simulink model "sim_discrete_planar_2dof"  (R2022b)
settings: Solver=VariableStepAuto  StartTime=0.0  StopTime=T_sim  FixedStep=param_global.Ta  RelTol=1e-3
InitFcn:
  | parameters_2dof;
undefined symbols (not defined in the file): coord_xaxis_len, coord_yaxis_len, coord_zaxis_len, fx, fy, fz, init_bus_param, param_global, param_path_set_points, param_robot, param_vis, path_coord_xaxis_len, path_coord_yaxis_len, path_coord_zaxis_len, path_param, q_0, q_0_p, tau_x, tau_y, tau_z, X, Y, Z
libraries: sm_lib x1241, nesl_utility x796
(part 34 of 34)

== matlab function sim_discrete_planar_2dof/Visualization q2/Spur Endeffektor/MATLAB Function ==
  settings: updateMethod=DISCRETE  sampleTime=param_global.Ta
  data: robot_model input; p1 output; p2 output; p3 output; p4 output; p5 output; p6 output; p7 output; p8 output; p9 output; p10 output; p11 output; p12 output; p13 output; p14 output; p15 output; p16 output; p17 output; p18 output; p19 output; p20 output; p21 output; p22 output; p23 output; p24 output; p25 output; p26 output; p27 output; p28 output; p29 output; p30 output; p31 output; p32 output; p33 output; p34 output; p35 output; p36 output; p37 output; p38 output; p39 output; p40 output; p41 output; p42 output; p43 output; p44 output; p45 output; p46 output; p47 output; p48 output; p49 output; p50 output; start input; delta input; p51 output; p52 output; p53 output; p54 output; p55 output; p56 output; p57 output; p58 output; p59 output; p60 output; p61 output; p62 output; p63 output; p64 output; p65 output; p66 output; p67 output; p68 output; p69 output; p70 output; p71 output; p72 output; p73 output; p74 output; p75 output; p76 output; p77 output; p78 output; p79 output; p80 output; p81 output; p82 output; p83 output; p84 output; p85 output; p86 output; p87 output; p88 output; p89 output; p90 output; p91 output; p92 output; p93 output; p94 output; p95 output; p96 output; p97 output; p98 output; p99 output; p100 output
function [p1,p2,p3,p4,p5,p6,p7,p8,p9,p10,p11,p12,p13,p14,p15,p16,p17,p18,p19,p20,p21,p22,p23,p24,p25,p26,p27,p28,p29,p30,p31,p32,p33,p34,p35,p36,p37,p38,p39,p40,p41,p42,p43,p44,p45,p46,p47,p48,p49,p50,p51,p52,p53,p54,p55,p56,p57,p58,p59,p60,p61,p62,p63,p64,p65,p66,p67,p68,p69,p70,p71,p72,p73,p74,p75,p76,p77,p78,p79,p80,p81,p82,p83,p84,p85,p86,p87,p88,p89,p90,p91,p92,p93,p94,p95,p96,p97,p98,p99,p100] = fcn(robot_model, start, delta)
persistent i t1 t2 t3 t4 t5 t6 t7 t8 t9 t10 t11 t12 t13 t14 t15 t16 t17 t18 t19 t20 t21 t22 t23 t24 t25 t26 t27 t28 t29 t30 t31 t32 t33 t34 t35 t36 t37 t38 t39 t40 t41 t42 t43 t44 t45 t46 t47 t48 t49 t50 t51 t52 t53 t54 t55 t56 t57 t58 t59 t60 t61 t62 t63 t64 t65 t66 t67 t68 t69 t70 t71 t72 t73 t74 t75 t76 t77 t78 t79 t80 t81 t82 t83 t84 t85 t86 t87 t88 t89 t90 t91 t92 t93 t94 t95 t96 t97 t98 t99 t100
if isempty(t1)
    i = 1;
    t1 = robot_model.H(1:3,4);
    t2 = t1;
    t3 = t1;
    t4 = t1;
    t5 = t1;
    t6 = t1;
    t7 = t1;
    t8 = t1;
    t9 = t1;
    t10 = t1;
    t11 = t1;
    t12 = t1;
    t13 = t1;
    t14 = t1;
    t15 = t1;
    t16 = t1;
    t17 = t1;
    t18 = t1;
    t19 = t1;
    t20 = t1;
    t21 = t1;
    t22 = t1;
    t23 = t1;
    t24 = t1;
    t25 = t1;
    t26 = t1;
    t27 = t1;
    t28 = t1;
    t29 = t1;
    t30 = t1;
    t31 = t1;
    t32 = t1;
    t33 = t1;
    t34 = t1;
    t35 = t1;
    t36 = t1;
    t37 = t1;
    t38 = t1;
    t39 = t1;
    t40 = t1;
    t41 = t1;
    t42 = t1;
    t43 = t1;
    t44 = t1;
    t45 = t1;
    t46 = t1;
    t47 = t1;
    t48 = t1;
    t49 = t1;
    t50 = t1;
    t51 = t1;
    t52 = t1;
    t53 = t1;
    t54 = t1;
    t55 = t1;
    t56 = t1;
    t57 = t1;
    t58 = t1;
    t59 = t1;
    t60 = t1;
    t61 = t1;
    t62 = t1;
    t63 = t1;
    t64 = t1;
    t65 = t1;
    t66 = t1;
    t67 = t1;
    t68 = t1;
    t69 = t1;
    t70 = t1;
    t71 = t1;
    t72 = t1;
    t73 = t1;
    t74 = t1;
    t75 = t1;
    t76 = t1;
    t77 = t1;
    t78 = t1;
    t79 = t1;
    t80 = t1;
    t81 = t1;
    t82 = t1;
    t83 = t1;
    t84 = t1;
    t85 = t1;
    t86 = t1;
    t87 = t1;
    t88 = t1;
    t89 = t1;
    t90 = t1;
    t91 = t1;
    t92 = t1;
    t93 = t1;
    t94 = t1;
    t95 = t1;
    t96 = t1;
    t97 = t1;
    t98 = t1;
    t99 = t1;
    t100 = t1;
    
end

switch i
    case start
        t1 = robot_model.H(1:3,4);
    case start+delta*1
        t2 = robot_model.H(1:3,4);
    case start+delta*2
        t3 = robot_model.H(1:3,4);
    case start+delta*3
        t4 = robot_model.H(1:3,4);
    case start+delta*4
        t5 = robot_model.H(1:3,4);
    case start+delta*5
        t6 = robot_model.H(1:3,4);
    case start+delta*6
        t7 = robot_model.H(1:3,4);
    case start+delta*7
        t8 = robot_model.H(1:3,4);
    case start+delta*8
        t9 = robot_model.H(1:3,4);
    case start+delta*9
        t10 = robot_model.H(1:3,4);
    case start+delta*10
        t11 = robot_model.H(1:3,4);
    case start+delta*11
        t12 = robot_model.H(1:3,4);
    case start+delta*12
        t13 = robot_model.H(1:3,4);
    case start+delta*13
        t14 = robot_model.H(1:3,4);
    case start+delta*14
        t15 = robot_model.H(1:3,4);
    case start+delta*15
        t16 = robot_model.H(1:3,4);
    case start+delta*16
        t17 = robot_model.H(1:3,4);
    case start+delta*17
        t18 = robot_model.H(1:3,4);
    case start+delta*18
        t19 = robot_model.H(1:3,4);
    case start+delta*19
        t20 = robot_model.H(1:3,4);
    case start+delta*20
        t21 = robot_model.H(1:3,4);
    case start+delta*21
        t22 = robot_model.H(1:3,4);
    case start+delta*22
        t23 = robot_model.H(1:3,4);
    case start+delta*23
        t24 = robot_model.H(1:3,4);
    case start+delta*24
        t25 = robot_model.H(1:3,4);
    case start+delta*25
        t26 = robot_model.H(1:3,4);
    case start+delta*26
        t27 = robot_model.H(1:3,4);
    case start+delta*27
        t28 = robot_model.H(1:3,4);
    case start+delta*28
        t29 = robot_model.H(1:3,4);
    case start+delta*29
        t30 = robot_model.H(1:3,4);
    case start+delta*30
        t31 = robot_model.H(1:3,4);
    case start+delta*31
        t32 = robot_model.H(1:3,4);
    case start+delta*32
        t33 = robot_model.H(1:3,4);
    case start+delta*33
        t34 = robot_model.H(1:3,4);
    case start+delta*34
        t35 = robot_model.H(1:3,4);
    case start+delta*35
        t36 = robot_model.H(1:3,4);
    case start+delta*36
        t37 = robot_model.H(1:3,4);
    case start+delta*37
        t38 = robot_model.H(1:3,4);
    case start+delta*38
        t39 = robot_model.H(1:3,4);
    case start+delta*39
        t40 = robot_model.H(1:3,4);
    case start+delta*40
        t41 = robot_model.H(1:3,4);
    case start+delta*41
        t42 = robot_model.H(1:3,4);
    case start+delta*42
        t43 = robot_model.H(1:3,4);
    case start+delta*43
        t44 = robot_model.H(1:3,4);
    case start+delta*44
        t45 = robot_model.H(1:3,4);
    case start+delta*45
        t46 = robot_model.H(1:3,4);
    case start+delta*46
        t47 = robot_model.H(1:3,4);
    case start+delta*47
        t48 = robot_model.H(1:3,4);
    case start+delta*48
        t49 = robot_model.H(1:3,4);
    case start+delta*49
        t50 = robot_model.H(1:3,4);
    case start+delta*50
        t51 = robot_model.H(1:3,4);
    case start+delta*51
        t52 = robot_model.H(1:3,4);
    case start+delta*52
        t53 = robot_model.H(1:3,4);
    case start+delta*53
        t54 = robot_model.H(1:3,4);
    case start+delta*54
        t55 = robot_model.H(1:3,4);
    case start+delta*55
        t56 = robot_model.H(1:3,4);
    case start+delta*56
        t57 = robot_model.H(1:3,4);
    case start+delta*57
        t58 = robot_model.H(1:3,4);
    case start+delta*58
        t59 = robot_model.H(1:3,4);
    case start+delta*59
        t60 = robot_model.H(1:3,4);
    case start+delta*60
        t61 = robot_model.H(1:3,4);
    case start+delta*61
        t62 = robot_model.H(1:3,4);
    case start+delta*62
        t63 = robot_model.H(1:3,4);
    case start+delta*63
        t64 = robot_model.H(1:3,4);
    case start+delta*64
        t65 = robot_model.H(1:3,4);
    case start+delta*65
        t66 = robot_model.H(1:3,4);
    case start+delta*66
        t67 = robot_model.H(1:3,4);
    case start+delta*67
        t68 = robot_model.H(1:3,4);
    case start+delta*68
        t69 = robot_model.H(1:3,4);
    case start+delta*69
        t70 = robot_model.H(1:3,4);
    case start+delta*70
        t71 = robot_model.H(1:3,4);
    case start+delta*71
        t72 = robot_model.H(1:3,4);
    case start+delta*72
        t73 = robot_model.H(1:3,4);
    case start+delta*73
        t74 = robot_model.H(1:3,4);
    case start+delta*74
        t75 = robot_model.H(1:3,4);
    case start+delta*75
        t76 = robot_model.H(1:3,4);
    case start+delta*76
        t77 = robot_model.H(1:3,4);
    case start+delta*77
        t78 = robot_model.H(1:3,4);
    case start+delta*78
        t79 = robot_model.H(1:3,4);
    case start+delta*79
        t80 = robot_model.H(1:3,4);
    case start+delta*80
        t81 = robot_model.H(1:3,4);
    case start+delta*81
        t82 = robot_model.H(1:3,4);
    case start+delta*82
        t83 = robot_model.H(1:3,4);
    case start+delta*83
        t84 = robot_model.H(1:3,4);
    case start+delta*84
        t85 = robot_model.H(1:3,4);
    case start+delta*85
        t86 = robot_model.H(1:3,4);
    case start+delta*86
        t87 = robot_model.H(1:3,4);
    case start+delta*87
        t88 = robot_model.H(1:3,4);
    case start+delta*88
        t89 = robot_model.H(1:3,4);
    case start+delta*89
        t90 = robot_model.H(1:3,4);
    case start+delta*90
        t91 = robot_model.H(1:3,4);
    case start+delta*91
        t92 = robot_model.H(1:3,4);
    case start+delta*92
        t93 = robot_model.H(1:3,4);
    case start+delta*93
        t94 = robot_model.H(1:3,4);
    case start+delta*94
        t95 = robot_model.H(1:3,4);
    case start+delta*95
        t96 = robot_model.H(1:3,4);
    case start+delta*96
        t97 = robot_model.H(1:3,4);
    case start+delta*97
        t98 = robot_model.H(1:3,4);
    case start+delta*98
        t99 = robot_model.H(1:3,4);
    case start+delta*99
        t100 = robot_model.H(1:3,4);
end

i = i+1;
p1 = t1;
p2 = t2;
p3 = t3;
p4 = t4;
p5 = t5;
p6 = t6;
p7 = t7;
p8 = t8;
p9 = t9;
p10 = t10;
p11 = t11;
p12 = t12;
p13 = t13;
p14 = t14;
p15 = t15;
p16 = t16;
p17 = t17;
p18 = t18;
p19 = t19;
p20 = t20;
p21 = t21;
p22 = t22;
p23 = t23;
p24 = t24;
p25 = t25;
p26 = t26;
p27 = t27;
p28 = t28;
p29 = t29;
p30 = t30;
p31 = t31;
p32 = t32;
p33 = t33;
p34 = t34;
p35 = t35;
p36 = t36;
p37 = t37;
p38 = t38;
p39 = t39;
p40 = t40;
p41 = t41;
p42 = t42;
p43 = t43;
p44 = t44;
p45 = t45;
p46 = t46;
p47 = t47;
p48 = t48;
p49 = t49;
p50 = t50;
p51 = t51;
p52 = t52;
p53 = t53;
p54 = t54;
p55 = t55;
p56 = t56;
p57 = t57;
p58 = t58;
p59 = t59;
p60 = t60;
p61 = t61;
p62 = t62;
p63 = t63;
p64 = t64;
p65 = t65;
p66 = t66;
p67 = t67;
p68 = t68;
p69 = t69;
p70 = t70;
p71 = t71;
p72 = t72;
p73 = t73;
p74 = t74;
p75 = t75;
p76 = t76;
p77 = t77;
p78 = t78;
p79 = t79;
p80 = t80;
p81 = t81;
p82 = t82;
p83 = t83;
p84 = t84;
p85 = t85;
p86 = t86;
p87 = t87;
p88 = t88;
p89 = t89;
p90 = t90;
p91 = t91;
p92 = t92;
p93 = t93;
p94 = t94;
p95 = t95;
p96 = t96;
p97 = t97;
p98 = t98;
p99 = t99;
p100 = t100;

== matlab function sim_discrete_planar_2dof/Debug Subsystem/manipulability ==
  settings: sampleTime=param_global.Ta
  data: robot_model input; w output
function w  = manipulability(robot_model)

J = robot_model.J(1:2,1:2);
det_J = det(J*J');
w = sqrt(abs(det_J));
%q = robot_model.q;
%qnorm = sum(q.^2);

== matlab function sim_discrete_planar_2dof/Visualization q2/dynamic single path visualize line (slow)/get poses ==
  settings: updateMethod=DISCRETE  sampleTime=path_display_update_time
  data: path_set input; p1 output; path_nr input; p2 output; p3 output; p4 output; p5 output; p6 output; p7 output; p8 output; p9 output; p10 output; param_path_set_points parameter
function [p1,p2,p3,p4,p5,p6,p7,p8,p9,p10] = fcn(path_set, path_nr, param_path_set_points)

if(path_set == 1)
    pose = param_path_set_points.paths_set1(:,:,path_nr);
elseif(path_set == 2)
    pose = param_path_set_points.paths_set2(:,:,path_nr);
else % path_set == 3
    pose = param_path_set_points.paths_set3(:,:,path_nr);
end

p1 = pose(1,:);
p2 = pose(2,:);
p3 = pose(3,:);
p4 = pose(4,:);
p5 = pose(5,:);
p6 = pose(6,:);
p7 = pose(7,:);
p8 = pose(8,:);
p9 = pose(9,:);
p10 = pose(10,:);

== matlab function sim_discrete_planar_2dof/Debug Subsystem/get pos ==
  settings: updateMethod=DISCRETE  sampleTime=param_global.Ta
  data: q input; yy_debug output Bus: yy_debug_bus; param_robot parameter; x_d_trj input Bus: x_d; init_bus_param parameter
function yy_debug  = fcn(q, param_robot, x_d_trj, init_bus_param)

yy_debug = init_bus_param.init_yy_debug_bus;

H = hom_transform_endeffector(q, param_robot);
y_t_W_E = H(1:3,4);
y_t_W_E_d = x_d_trj.p_d;

R_W_E = H(1:3,1:3);
ori_t_W_E = rotm2eul(R_W_E, 'XYZ');
ori_t_W_E_d = rotm2eul(quaternion2rotation(x_d_trj.q_d), 'XYZ');

yy_debug.x =  y_t_W_E(1);
yy_debug.x_d = y_t_W_E_d(1);

yy_debug.y =  y_t_W_E(2);
yy_debug.y_d = y_t_W_E_d(2);

yy_debug.z =  y_t_W_E(3);
yy_debug.z_d = y_t_W_E_d(3);

yy_debug.phi = ori_t_W_E(1);
yy_debug.phi_d = ori_t_W_E_d(1);

yy_debug.theta = ori_t_W_E(2);
yy_debug.theta_d = ori_t_W_E_d(2);

yy_debug.psi = ori_t_W_E(3);
yy_debug.psi_d = ori_t_W_E_d(3);

quat_err = quat_mult(rotation2quaternion(R_W_E), quat_inv(x_d_trj.q_d));
yy_debug.rot_x_err = quat_err(2);
yy_debug.rot_y_err = quat_err(3);
yy_debug.rot_z_err = quat_err(4);

== matlab function sim_discrete_planar_2dof/Debug Subsystem/MATLAB Function1 ==
  data: debug_data input Bus: debug_data_bus; e_x output; e_y output; e_x_p output; e_y_p output; e_x_pp output; e_y_pp output; x_d_trj input Bus: x_d
function [e_x, e_y, e_x_p, e_y_p, e_x_pp, e_y_pp] = fcn(debug_data, x_d_trj)

p    = debug_data.p;
p_p  = debug_data.p_p;
p_pp = debug_data.p_pp;

p_d    = x_d_trj.p_d;
p_d_p  = x_d_trj.p_d_p;
p_d_pp = x_d_trj.p_d_pp;

e_x    = p_d(1)    - p(1);
e_x_p  = p_d_p(1)  - p_p(1);
e_x_pp = p_d_pp(1) - p_pp(1);

e_y    = p_d(2)    - p(2);
e_y_p  = p_d_p(2)  - p_p(2);
e_y_pp = p_d_pp(2) - p_pp(2);

== matlab function sim_discrete_planar_2dof/Simulation models/Robot model/Reduziertes System ==
  data: tau input; q_pp output; q input; q_p input; param_robot parameter
function q_pp =fcn(tau, q, q_p, param_robot)

M=inertia_matrix(q,param_robot);                          % rigid body inertia matrix
C=coriolis_matrix(q,q_p,param_robot);                     % coriolis matrix
g=gravitational_forces(q,param_robot);                    % gravitational forces

q_pp = M\(tau - C*q_p - g);

% q_pp = M\(tau-C*q_p-g - 0.5*diag(ones(7,1))*q_p(3:9)); % mit Reibung

== matlab function sim_discrete_planar_2dof/Visualization q2/dynamic single path visualize line (slow)/get poses1 ==
  settings: updateMethod=DISCRETE  sampleTime=path_display_update_time
  data: path_set input; p1 output; path_nr input; p2 output; p3 output; p4 output; p5 output; p6 output; p7 output; p8 output; p9 output; p10 output; param_path_set_points parameter
function [p1,p2,p3,p4,p5,p6,p7,p8,p9,p10] = fcn(path_set, path_nr, param_path_set_points)

if(path_set == 1)
    pose = param_path_set_points.paths_set1(:,:,path_nr);
elseif(path_set == 2)
    pose = param_path_set_points.paths_set2(:,:,path_nr);
else % path_set == 3
    pose = param_path_set_points.paths_set3(:,:,path_nr);
end

p1 = pose(1,:);
p2 = pose(2,:);
p3 = pose(3,:);
p4 = pose(4,:);
p5 = pose(5,:);
p6 = pose(6,:);
p7 = pose(7,:);
p8 = pose(8,:);
p9 = pose(9,:);
p10 = pose(10,:);

== matlab function sim_discrete_planar_2dof/External force subsystem/external_force ==
  settings: updateMethod=DISCRETE  sampleTime=param_global.Ta
  data: f_W_E input; robot_model input; debug_snap_data input Bus: debug_snap_data_bus; tau_ext output; mode input; f_ext output
function [tau_ext, f_ext] = fcn(f_W_E, robot_model, debug_snap_data, mode)
    if(mode == 1) % Kraft ist im Weltsystem definiert
        f_ext = f_W_E; %Externe Kraft / Moment in Welt KOS definiert
    elseif(mode == 2) % Externe Kraft / Moment in Endeffektor KOS definiert
        % Rechnet die Kräfte im Weltsystem die auf den Endeffektor um in Kräfte,
        % die im Endeffektorsystem direkt auf den Endeffektor wirken, damit eine
        % intuitiverer Steuerung möglich ist (Der Benutzer legt ja auch die Kräfte
        % aus Sicht des Endeffektorsystem an und nicht aus Sicht des Weltsystems)
        
        R_W_E = robot_model.H(1:3,1:3);
        
        % indizes nicht ganz logisch
        F_W_E = f_W_E(1:3);
        tau_W_E = f_W_E(4:6);
        
        
        F_E_E = R_W_E*F_W_E;
        tau_E_E = R_W_E*tau_W_E;
        
        f_ext = [F_E_E;tau_E_E];
    else % Externe Kraft im Pfadkoordinatensystem des aktiven Pfads
        F_W_E = f_W_E(1:3);
        tau_W_E = f_W_E(4:6);
        active_path_index_t = debug_snap_data.active_path_index_t;
        if(active_path_index_t == 0) % if CT CONTROL TODO: Problem bei orienation snap wenn path index > N_path ist.
            R_W_path = eye(3);
        else
            R_W_path = debug_snap_data.Rot_transport_frame(:,:,active_path_index_t); % only active path
        end

        F_path_E = R_W_path*F_W_E;
        tau_path_E = R_W_path*tau_W_E;
        
        f_ext = [F_path_E;tau_path_E];
    end
    %theta_star = debug_snap_data.theta_star;
    %N_path = param_global.N_path;

    %R_W_path = debug_snap_data.Rot_transport_frame; % all paths
    %path_frame_position = zeros(3,2,N_path);
    %for i=1:1:N_path
    %    [sigma, ~, ~, ~] = eval_path_t(theta_star(i), i, path_param);
    %    path_frame_position(:,:,i) = [sigma rotm2eul(R_W_path(:,:,i), 'XYZ')];
    %end

    J = robot_model.J;
    tau_ext_temp = J' * f_ext;
    tau_ext = tau_ext_temp(3:9);
end

== matlab function sim_discrete_planar_2dof/Simulation models/controller/Controller Enable ==
  settings: sampleTime=param_global.Ta
  data: en_arr output; selector input uint32
function en_arr  = fcn(selector)
    % Wichtig: selector \in [1,max(selector)]
    % Es wird ein bool array ausgegeben, welches nur den index 1 setzt, der
    % mit selector übereinstimmt. Achtung: Size von "en_arr" muss
    % entsprechend der Ausgänge unter "Edit Data" manuell angegeben werden.
    % Beispiel: 4 Inputs, max(selector)=4, Size = [1 4]
    % z. B. selector = 2
    % 1:4 == selector liefert [0 1 0 0]
    en_arr = (1:6 == selector)';
end

== matlab function sim_discrete_planar_2dof/Visualization q2/dynamic single path visualize line (slow)/get poses2 ==
  settings: updateMethod=DISCRETE  sampleTime=path_display_update_time
  data: path_set input; p1 output; path_nr input; p2 output; p3 output; p4 output; p5 output; p6 output; p7 output; p8 output; p9 output; p10 output; param_path_set_points parameter
function [p1,p2,p3,p4,p5,p6,p7,p8,p9,p10] = fcn(path_set, path_nr, param_path_set_points)

if(path_set == 1)
    pose = param_path_set_points.paths_set1(:,:,path_nr);
elseif(path_set == 2)
    pose = param_path_set_points.paths_set2(:,:,path_nr);
else % path_set == 3
    pose = param_path_set_points.paths_set3(:,:,path_nr);
end

p1 = pose(1,:);
p2 = pose(2,:);
p3 = pose(3,:);
p4 = pose(4,:);
p5 = pose(5,:);
p6 = pose(6,:);
p7 = pose(7,:);
p8 = pose(8,:);
p9 = pose(9,:);
p10 = pose(10,:);

== matlab function sim_discrete_planar_2dof/Visualization q2/dynamic single path visualize line (slow)/get poses3 ==
  settings: updateMethod=DISCRETE  sampleTime=path_display_update_time
  data: path_set input; p1 output; path_nr input; p2 output; p3 output; p4 output; p5 output; p6 output; p7 output; p8 output; p9 output; p10 output; param_path_set_points parameter
function [p1,p2,p3,p4,p5,p6,p7,p8,p9,p10] = fcn(path_set, path_nr, param_path_set_points)

if(path_set == 1)
    pose = param_path_set_points.paths_set1(:,:,path_nr);
elseif(path_set == 2)
    pose = param_path_set_points.paths_set2(:,:,path_nr);
else % path_set == 3
    pose = param_path_set_points.paths_set3(:,:,path_nr);
end

p1 = pose(11,:);
p2 = pose(12,:);
p3 = pose(13,:);
p4 = pose(14,:);
p5 = pose(15,:);
p6 = pose(16,:);
p7 = pose(17,:);
p8 = pose(18,:);
p9 = pose(19,:);
p10 = pose(20,:);

== matlab function sim_discrete_planar_2dof/External force subsystem/auto force functions/get signals ==
  data: t input; y output size=6; t0 input; y0 input; m0 input; custom_mode output
function [y,custom_mode] = fcn(t, t0, y0, m0)
% ti_a ... Anfangszeit
% ti_e ... Endzeit
% yi ... Pulswert
% mi ... mode: 1 if f_w_e, 2 if f_e_e, 3 if f_path
% outputs
% y ... chosen Pulswert
% custom mode: chose mode

i = sum(t >= t0);
y = y0(i,:)';
custom_mode = m0(i);

== matlab function sim_discrete_planar_2dof/Visualization q2/show pritzi force arrows/MATLAB Function1 ==
  data: q input; px output; py output; pz output; f_ext input; param_robot parameter
function [px, py, pz] = fcn(q, f_ext, param_robot)
    %J=geo_jacobian_endeffector(q,param_robot);
    H=hom_transform_endeffector(q,param_robot);

    if norm(f_ext) < 1e-3
        px = 0;
        py = 0;
        pz = 0;
    else
        p = H(1:3, 4);
        px = p(1);
        py = p(2);
        pz = p(3);
    end
end

== matlab function sim_discrete_planar_2dof/Visualization q2/show pritzi force arrows/MATLAB Function2 ==
  data: q input; px output; py output; pz output; f_ext input; param_robot parameter
function [px, py, pz] = fcn(q, f_ext, param_robot)
    %J=geo_jacobian_endeffector(q,param_robot);
    H=hom_transform_endeffector(q,param_robot);

    if norm(f_ext) < 1e-3
        px = 0;
        py = 0;
        pz = 0;
    else
        p = H(1:3, 4);
        px = p(1);
        py = p(2);
        pz = p(3);
    end
end

== matlab function sim_discrete_planar_2dof/Visualization q2/show pritzi force arrows/calc_rotations ==
  data: fx input; Rx output; Ry output; Rz output; fy input; fz input
function [Rx, Ry, Rz] = fcn(fx, fy, fz)
    Rx = -atan2(fy, fz);
    Ry = atan2(fx, sqrt(fy^2 + fz^2));
    Rz = 0;
end

== matlab function sim_discrete_planar_2dof/Visualization q2/show pritzi force arrows/calc_rotations1 ==
  data: taux input; Rx output; Ry output; Rz output; tauy input; tauz input
function [Rx, Ry, Rz] = fcn(taux, tauy, tauz)
    Rx = -atan2(tauy, tauz);
    Ry = atan2(taux, sqrt(tauy^2 + tauz^2));
    Rz = 0;
end

== matlab function sim_discrete_planar_2dof/External force subsystem/auto force functions/get signals1 ==
  data: t input; y output size=6; t0_a input; t0_e input; y0 input; m0 input; t1_a input; t1_e input; y1 input; m1 input; t2_a input; t2_e input; y2 input; m2 input; t3_a input; t3_e input; y3 input; m3 input; t4_a input; t4_e input; y4 input; custom_mode output; m4 input
function [y,custom_mode] = fcn(t, t0_a, t0_e, y0, m0, t1_a, t1_e, y1, m1, t2_a, t2_e, y2, m2, t3_a, t3_e, y3, m3, t4_a, t4_e, y4, m4)
% ti_a ... Anfangszeit
% ti_e ... Endzeit
% yi ... Pulswert
% mi ... mode: 1 if f_w_e, 2 if f_e_e, 3 if f_path
% outputs
% y ... chosen Pulswert
% custom mode: chose mode

if(t >= t0_a && t < t0_e)
    y = y0;
    custom_mode = m0; % f_path
elseif(t >= t1_a && t < t1_e)
    y = y1;
    custom_mode = m1; % f_e_e
elseif(t >= t2_a && t < t2_e)
    y = y2;
    custom_mode = m2; % f_path
elseif(t >= t3_a && t < t3_e)
    y = y3;
    custom_mode = m3; % f_path
elseif(t >= t4_a && t < t4_e)
    y = y4;
    custom_mode = m4; % f_path
else
    y = [0 0 0 0 0 0]';
    custom_mode = 1; % f_w_e
end

== matlab function sim_discrete_planar_2dof/External force subsystem/auto force functions/get signals2 ==
  data: t input; y output size=6; t0 input; y0 input; m0 input; custom_mode output
function [y,custom_mode] = fcn(t, t0, y0, m0)
% ti_a ... Anfangszeit
% ti_e ... Endzeit
% yi ... Pulswert
% mi ... mode: 1 if f_w_e, 2 if f_e_e, 3 if f_path
% outputs
% y ... chosen Pulswert
% custom mode: chose mode

i = sum(t >= t0);
y = y0(i,:)';
custom_mode = m0(i);

== matlab function sim_discrete_planar_2dof/Visualization q2/dynamic single path visualize spline (slow)/get poses ==
  settings: updateMethod=DISCRETE  sampleTime=path_display_update_time
  data: path_set input; p1 output; path_nr input; p2 output; p3 output; p4 output; p5 output; p6 output; p7 output; p8 output; p9 output; p10 output; param_path_set_points parameter
function [p1,p2,p3,p4,p5,p6,p7,p8,p9,p10] = fcn(path_set, path_nr, param_path_set_points)

if(path_set == 1)
    pose = param_path_set_points.paths_set1(:,:,path_nr);
elseif(path_set == 2)
    pose = param_path_set_points.paths_set2(:,:,path_nr);
else % path_set == 3
    pose = param_path_set_points.paths_set3(:,:,path_nr);
end

p1 = pose(1,:);
p2 = pose(2,:);
p3 = pose(3,:);
p4 = pose(4,:);
p5 = pose(5,:);
p6 = pose(6,:);
p7 = pose(7,:);
p8 = pose(8,:);
p9 = pose(9,:);
p10 = pose(10,:);

== matlab function sim_discrete_planar_2dof/Visualization q2/dynamic single path visualize spline (slow)/get poses1 ==
  settings: updateMethod=DISCRETE  sampleTime=path_display_update_time
  data: path_set input; p1 output; path_nr input; p2 output; p3 output; p4 output; p5 output; p6 output; p7 output; p8 output; p9 output; p10 output; param_path_set_points parameter
function [p1,p2,p3,p4,p5,p6,p7,p8,p9,p10] = fcn(path_set, path_nr, param_path_set_points)

if(path_set == 1)
    pose = param_path_set_points.paths_set1(:,:,path_nr);
elseif(path_set == 2)
    pose = param_path_set_points.paths_set2(:,:,path_nr);
else % path_set == 3
    pose = param_path_set_points.paths_set3(:,:,path_nr);
end

p1 = pose(1,:);
p2 = pose(2,:);
p3 = pose(3,:);
p4 = pose(4,:);
p5 = pose(5,:);
p6 = pose(6,:);
p7 = pose(7,:);
p8 = pose(8,:);
p9 = pose(9,:);
p10 = pose(10,:);

== matlab function sim_discrete_planar_2dof/Visualization q2/dynamic single path visualize spline (slow)/get poses2 ==
  settings: updateMethod=DISCRETE  sampleTime=path_display_update_time
  data: path_set input; p1 output; path_nr input; p2 output; p3 output; p4 output; p5 output; p6 output; p7 output; p8 output; p9 output; p10 output; param_path_set_points parameter
function [p1,p2,p3,p4,p5,p6,p7,p8,p9,p10] = fcn(path_set, path_nr, param_path_set_points)

if(path_set == 1)
    pose = param_path_set_points.paths_set1(:,:,path_nr);
elseif(path_set == 2)
    pose = param_path_set_points.paths_set2(:,:,path_nr);
else % path_set == 3
    pose = param_path_set_points.paths_set3(:,:,path_nr);
end

p1 = pose(1,:);
p2 = pose(2,:);
p3 = pose(3,:);
p4 = pose(4,:);
p5 = pose(5,:);
p6 = pose(6,:);
p7 = pose(7,:);
p8 = pose(8,:);
p9 = pose(9,:);
p10 = pose(10,:);

== matlab function sim_discrete_planar_2dof/Simulation models/Robot model bus ==
  settings: updateMethod=DISCRETE  sampleTime=param_global.Ta
  data: q input; q_p input; q_pp input; robot_model output Bus: robot_model; param_robot parameter
function robot_model = fcn(q, q_p, q_pp, param_robot)
% Parameters
robot_model=struct();
robot_model.q=q;                    % generalized coordinates
robot_model.q_p=q_p;                % time derivative of generalized coordinates
robot_model.q_pp=q_pp;
robot_model.H=hom_transform_endeffector(q, param_robot);            % homogenous transformation to end-effector
robot_model.J=geo_jacobian_endeffector(q,param_robot);              % geometric jacobian to end-effector
robot_model.J_p=geo_jacobian_endeffector_p(q, q_p,param_robot);     % time derivative of geometric jacobian to end-effector
robot_model.M=inertia_matrix(q,param_robot);                        % inertia matrix
robot_model.C=coriolis_matrix(q, q_p,param_robot);                  % coriolis matrix
robot_model.g=gravitational_forces(q,param_robot);                  % gravitational forces
end

== matlab function sim_discrete_planar_2dof/Visualization q2/dynamic single path visualize spline (slow)/get poses3 ==
  settings: updateMethod=DISCRETE  sampleTime=path_display_update_time
  data: path_set input; p1 output; path_nr input; p2 output; p3 output; p4 output; p5 output; p6 output; p7 output; p8 output; p9 output; p10 output; param_path_set_points parameter
function [p1,p2,p3,p4,p5,p6,p7,p8,p9,p10] = fcn(path_set, path_nr, param_path_set_points)

if(path_set == 1)
    pose = param_path_set_points.paths_set1(:,:,path_nr);
elseif(path_set == 2)
    pose = param_path_set_points.paths_set2(:,:,path_nr);
else % path_set == 3
    pose = param_path_set_points.paths_set3(:,:,path_nr);
end

p1 = pose(11,:);
p2 = pose(12,:);
p3 = pose(13,:);
p4 = pose(14,:);
p5 = pose(15,:);
p6 = pose(16,:);
p7 = pose(17,:);
p8 = pose(18,:);
p9 = pose(19,:);
p10 = pose(20,:);

== matlab function sim_discrete_planar_2dof/tic-toc/MATLAB Function ==
  settings: updateMethod=DISCRETE  sampleTime=param_global.Ta
  data: t input; param_vis parameter
function fcn(t, param_vis)

if(t==0)
    tic;
end

if(t==param_vis.T)
    toc;
end

== matlab function sim_discrete_planar_2dof/Debug Subsystem/messages on end/MATLAB Function ==
  settings: updateMethod=DISCRETE  sampleTime=param_global.Ta
  data: t input; param_vis parameter
function fcn(t, param_vis)

if(t==0)
    tic;
end

if(t==param_vis.T)
    toc;
end

== matlab function sim_discrete_planar_2dof/tic-toc/tic-toc/MATLAB Function ==
  settings: updateMethod=DISCRETE
function fcn()
persistent flag;

if(isempty(flag))
    flag=0;
end

if(flag == 0)
    tic
    flag=1;
else
    disp(['Simulink execution time: ', sprintf('%f', toc), ' s']);
end

== matlab function sim_discrete_planar_2dof/Debug Subsystem/messages on end/output debug text/MATLAB Function ==
  settings: updateMethod=DISCRETE
  data: x_err_mean input; y_err_mean input
function fcn(x_err_mean, y_err_mean)
    disp(['mean x_err = ', sprintf('%g', x_err_mean), ' m']);
    disp(['mean y_err = ', sprintf('%g', y_err_mean), ' m']);
end

== matlab function sim_discrete_planar_2dof/Debug Subsystem/MATLAB Function ==
  data: x_err_mean_out output; x_err input; y_err input; y_err_mean_out output
function [x_err_mean_out, y_err_mean_out] = fcn(x_err, y_err)

persistent cnt;
persistent x_err_mean;
persistent y_err_mean;

if(isempty(cnt))
    cnt = 1;
    x_err_mean = 0;
    y_err_mean = 0;
end
% m1 =  a          = (0*m0+a)/1
% m2 = (a+b)/2     = (1*m1+b)/2
% m3 = (a+b+c)/3   = (2*m2+c)/3
% m4 = (a+b+c+d)/4 = (3*m3+d)/4
% ...
% mi = ((i-1)*m_{i-1})/i; 
x_err_mean = ((cnt-1)*x_err_mean + x_err)/cnt;
y_err_mean = ((cnt-1)*y_err_mean + y_err)/cnt;

x_err_mean_out = x_err_mean;
y_err_mean_out = y_err_mean;

cnt = cnt + 1;

%Die iterative Methode ist anfälliger für Rundungsfehler.
%Die iterative Methode kann bei sehr großen Datenmengen numerisch instabil
% werden
